simulink model "SOC_2023b"  (R2023b)
settings: Solver=VariableStepAuto  StartTime=0.0  StopTime=10.0  RelTol=1e-3
undefined symbols (not defined in the file or in its folder): Initial_SOC
(part 10 of 187)

== system SOC_2023b/SOC/Multi-Temperature SOC EKF5/Extended Kalman Filter - Multi Temperature EKF ==
  note: McMaster Automotive Research Centre - MARC
      | Electrical Engineer: Romulo Navega Vieira, M.Sc and MBA - PhD Candidate
  Current : Inport  Partition=on  PartitionDimension=1  PartitionWidth=1  PartitionOffset=0
  Voltage : Inport  Port=2  Partition=on  PartitionDimension=1  PartitionWidth=1  PartitionOffset=0
  Temperature : Inport  Port=3  Partition=on  PartitionDimension=1  PartitionWidth=1  PartitionOffset=0
  Constant{1..7} (7 alike) : Constant  Value=[0;0;0]
  Delay{1..2,5,7,9,11,13} (7 alike) : Delay  DelayLength=1  InitialConditionSource=Input port  InitialCondition=[0;0;0;Initial_SOC]
      settings: InputPortMap=u0,p4
  Delay{3..4,6,8,10,12,14} (7 alike) : Delay  DelayLength=1  InitialCondition=diag([1 1 1 0.01])
  EKF- 40deg C : MATLAB Function  TreatAsAtomicUnit=on
  EKF- 40deg C1 : MATLAB Function  Commented=on  TreatAsAtomicUnit=on
  EKF- 40deg C2 : MATLAB Function  Commented=on  TreatAsAtomicUnit=on
  EKF- 40deg C3 : MATLAB Function  Commented=on  TreatAsAtomicUnit=on
  EKF- 40deg C4 : MATLAB Function  Commented=on  TreatAsAtomicUnit=on
  EKF- 40deg C5 : MATLAB Function  Commented=on  TreatAsAtomicUnit=on
  EKF- 40deg C6 : MATLAB Function  Commented=on  TreatAsAtomicUnit=on
  For Each : ForEach  DisableCoverage=on
  MATLAB Function : MATLAB Function  TreatAsAtomicUnit=on
  MATLAB Function1 : MATLAB Function  TreatAsAtomicUnit=on
  MATLAB Function2 : MATLAB Function  TreatAsAtomicUnit=on
  MATLAB Function3 : MATLAB Function  TreatAsAtomicUnit=on
  MATLAB Function4 : MATLAB Function  TreatAsAtomicUnit=on
  MATLAB Function5 : MATLAB Function  TreatAsAtomicUnit=on
  MATLAB Function6 : MATLAB Function  TreatAsAtomicUnit=on
  MATLAB Function7 : MATLAB Function  TreatAsAtomicUnit=on
  Vector Concatenate{,1..6} (7 alike) : Concatenate
  SOC : Outport  ConcatenationDimension=1
  wires:
  Voltage/1 -> EKF- 40deg C1/2(Voltage), EKF- 40deg C2/2(Voltage), EKF- 40deg C3/2(Voltage), EKF- 40deg C4/2(Voltage), EKF- 40deg C5/2(Voltage), EKF- 40deg C6/2(Voltage), MATLAB Function7/1(Voltage), MATLAB Function6/1(Voltage), MATLAB Function5/1(Voltage), MATLAB Function4/1(Voltage), MATLAB Function3/1(Voltage), MATLAB Function2/1(Voltage), MATLAB Function/1(Voltage), EKF- 40deg C/2(Voltage)
  MATLAB Function/1(Initial_SOC) -> Vector Concatenate/2
  EKF- 40deg C4/1(zSOC) -> MATLAB Function1/6(SOC0)
  Delay14/1 -> EKF- 40deg C6/4(P)
  EKF- 40deg C3/2(xf) -> Delay7/1
  Vector Concatenate1/1 -> Delay1/2
  EKF- 40deg C6/1(zSOC) -> MATLAB Function1/8(SOCn20)
  EKF- 40deg C6/2(xf) -> Delay13/1
  Vector Concatenate3/1 -> Delay7/2
  MATLAB Function7/1(Initial_SOC) -> Vector Concatenate6/2
  Vector Concatenate2/1 -> Delay5/2
  EKF- 40deg C5/1(zSOC) -> MATLAB Function1/7(SOCn10)
  Constant7/1 -> Vector Concatenate6/1
  Vector Concatenate5/1 -> Delay11/2
  EKF- 40deg C1/3(P1) -> Delay4/1
  Constant6/1 -> Vector Concatenate5/1
  EKF- 40deg C6/3(P1) -> Delay14/1
  MATLAB Function1/1(SOCF) -> SOC/1
  Current/1 -> EKF- 40deg C6/1(Current), EKF- 40deg C5/1(Current), EKF- 40deg C4/1(Current), EKF- 40deg C3/1(Current), EKF- 40deg C2/1(Current), EKF- 40deg C1/1(Current), EKF- 40deg C/1(Current)
  EKF- 40deg C4/3(P1) -> Delay10/1
  Delay11/1 -> EKF- 40deg C5/3(x)
  Delay13/1 -> EKF- 40deg C6/3(x)
  MATLAB Function5/1(Initial_SOC) -> Vector Concatenate4/2
  MATLAB Function6/1(Initial_SOC) -> Vector Concatenate5/2
  EKF- 40deg C5/2(xf) -> Delay11/1
  Delay10/1 -> EKF- 40deg C4/4(P)
  EKF- 40deg C5/3(P1) -> Delay12/1
  Constant5/1 -> Vector Concatenate4/1
  Delay2/1 -> EKF- 40deg C/3(x)
  EKF- 40deg C4/2(xf) -> Delay9/1
  EKF- 40deg C3/1(zSOC) -> MATLAB Function1/5(SOC10)
  EKF- 40deg C2/3(P1) -> Delay6/1
  EKF- 40deg C2/1(zSOC) -> MATLAB Function1/4(SOC20)
  Vector Concatenate6/1 -> Delay13/2
  Constant4/1 -> Vector Concatenate3/1
  Temperature/1 -> MATLAB Function1/1(Temp)
  EKF- 40deg C2/2(xf) -> Delay5/1
  Delay7/1 -> EKF- 40deg C3/3(x)
  Vector Concatenate/1 -> Delay2/2
  Delay12/1 -> EKF- 40deg C5/4(P)
  Vector Concatenate4/1 -> Delay9/2
  EKF- 40deg C1/1(zSOC) -> MATLAB Function1/3(SOC30)
  Delay5/1 -> EKF- 40deg C2/3(x)
  MATLAB Function4/1(Initial_SOC) -> Vector Concatenate3/2
  Delay6/1 -> EKF- 40deg C2/4(P)
  EKF- 40deg C/2(xf) -> Delay2/1
  Delay3/1 -> EKF- 40deg C/4(P)
  EKF- 40deg C3/3(P1) -> Delay8/1
  Delay9/1 -> EKF- 40deg C4/3(x)
  Delay8/1 -> EKF- 40deg C3/4(P)
  Constant3/1 -> Vector Concatenate2/1
  MATLAB Function2/1(Initial_SOC) -> Vector Concatenate1/2
  EKF- 40deg C/1(zSOC) -> MATLAB Function1/2(SOC40)
  MATLAB Function3/1(Initial_SOC) -> Vector Concatenate2/2
  Constant2/1 -> Vector Concatenate1/1
  Constant1/1 -> Vector Concatenate/1
  Delay4/1 -> EKF- 40deg C1/4(P)
  EKF- 40deg C/3(P1) -> Delay3/1
  Delay1/1 -> EKF- 40deg C1/3(x)
  EKF- 40deg C1/2(xf) -> Delay1/1

== system SOC_2023b/SOC/Multi-Temperature SOC EKF50/Extended Kalman Filter - Multi Temperature EKF ==
  note: McMaster Automotive Research Centre - MARC
      | Electrical Engineer: Romulo Navega Vieira, M.Sc and MBA - PhD Candidate
  Current : Inport  Partition=on  PartitionDimension=1  PartitionWidth=1  PartitionOffset=0
  Voltage : Inport  Port=2  Partition=on  PartitionDimension=1  PartitionWidth=1  PartitionOffset=0
  Temperature : Inport  Port=3  Partition=on  PartitionDimension=1  PartitionWidth=1  PartitionOffset=0
  Constant{1..7} (7 alike) : Constant  Value=[0;0;0]
  Delay{1..2,5,7,9,11,13} (7 alike) : Delay  DelayLength=1  InitialConditionSource=Input port  InitialCondition=[0;0;0;Initial_SOC]
      settings: InputPortMap=u0,p4
  Delay{3..4,6,8,10,12,14} (7 alike) : Delay  DelayLength=1  InitialCondition=diag([1 1 1 0.01])
  EKF- 40deg C : MATLAB Function  TreatAsAtomicUnit=on
  EKF- 40deg C1 : MATLAB Function  Commented=on  TreatAsAtomicUnit=on
  EKF- 40deg C2 : MATLAB Function  Commented=on  TreatAsAtomicUnit=on
  EKF- 40deg C3 : MATLAB Function  Commented=on  TreatAsAtomicUnit=on
  EKF- 40deg C4 : MATLAB Function  Commented=on  TreatAsAtomicUnit=on
  EKF- 40deg C5 : MATLAB Function  Commented=on  TreatAsAtomicUnit=on
  EKF- 40deg C6 : MATLAB Function  Commented=on  TreatAsAtomicUnit=on
  For Each : ForEach  DisableCoverage=on
  MATLAB Function : MATLAB Function  TreatAsAtomicUnit=on
  MATLAB Function1 : MATLAB Function  TreatAsAtomicUnit=on
  MATLAB Function2 : MATLAB Function  TreatAsAtomicUnit=on
  MATLAB Function3 : MATLAB Function  TreatAsAtomicUnit=on
  MATLAB Function4 : MATLAB Function  TreatAsAtomicUnit=on
  MATLAB Function5 : MATLAB Function  TreatAsAtomicUnit=on
  MATLAB Function6 : MATLAB Function  TreatAsAtomicUnit=on
  MATLAB Function7 : MATLAB Function  TreatAsAtomicUnit=on
  Vector Concatenate{,1..6} (7 alike) : Concatenate
  SOC : Outport  ConcatenationDimension=1
  wires:
  Voltage/1 -> EKF- 40deg C1/2(Voltage), EKF- 40deg C2/2(Voltage), EKF- 40deg C3/2(Voltage), EKF- 40deg C4/2(Voltage), EKF- 40deg C5/2(Voltage), EKF- 40deg C6/2(Voltage), MATLAB Function7/1(Voltage), MATLAB Function6/1(Voltage), MATLAB Function5/1(Voltage), MATLAB Function4/1(Voltage), MATLAB Function3/1(Voltage), MATLAB Function2/1(Voltage), MATLAB Function/1(Voltage), EKF- 40deg C/2(Voltage)
  MATLAB Function/1(Initial_SOC) -> Vector Concatenate/2
  EKF- 40deg C4/1(zSOC) -> MATLAB Function1/6(SOC0)
  Delay14/1 -> EKF- 40deg C6/4(P)
  EKF- 40deg C3/2(xf) -> Delay7/1
  Vector Concatenate1/1 -> Delay1/2
  EKF- 40deg C6/1(zSOC) -> MATLAB Function1/8(SOCn20)
  EKF- 40deg C6/2(xf) -> Delay13/1
  Vector Concatenate3/1 -> Delay7/2
  MATLAB Function7/1(Initial_SOC) -> Vector Concatenate6/2
  Vector Concatenate2/1 -> Delay5/2
  EKF- 40deg C5/1(zSOC) -> MATLAB Function1/7(SOCn10)
  Constant7/1 -> Vector Concatenate6/1
  Vector Concatenate5/1 -> Delay11/2
  EKF- 40deg C1/3(P1) -> Delay4/1
  Constant6/1 -> Vector Concatenate5/1
  EKF- 40deg C6/3(P1) -> Delay14/1
  MATLAB Function1/1(SOCF) -> SOC/1
  Current/1 -> EKF- 40deg C6/1(Current), EKF- 40deg C5/1(Current), EKF- 40deg C4/1(Current), EKF- 40deg C3/1(Current), EKF- 40deg C2/1(Current), EKF- 40deg C1/1(Current), EKF- 40deg C/1(Current)
  EKF- 40deg C4/3(P1) -> Delay10/1
  Delay11/1 -> EKF- 40deg C5/3(x)
  Delay13/1 -> EKF- 40deg C6/3(x)
  MATLAB Function5/1(Initial_SOC) -> Vector Concatenate4/2
  MATLAB Function6/1(Initial_SOC) -> Vector Concatenate5/2
  EKF- 40deg C5/2(xf) -> Delay11/1
  Delay10/1 -> EKF- 40deg C4/4(P)
  EKF- 40deg C5/3(P1) -> Delay12/1
  Constant5/1 -> Vector Concatenate4/1
  Delay2/1 -> EKF- 40deg C/3(x)
  EKF- 40deg C4/2(xf) -> Delay9/1
  EKF- 40deg C3/1(zSOC) -> MATLAB Function1/5(SOC10)
  EKF- 40deg C2/3(P1) -> Delay6/1
  EKF- 40deg C2/1(zSOC) -> MATLAB Function1/4(SOC20)
  Vector Concatenate6/1 -> Delay13/2
  Constant4/1 -> Vector Concatenate3/1
  Temperature/1 -> MATLAB Function1/1(Temp)
  EKF- 40deg C2/2(xf) -> Delay5/1
  Delay7/1 -> EKF- 40deg C3/3(x)
  Vector Concatenate/1 -> Delay2/2
  Delay12/1 -> EKF- 40deg C5/4(P)
  Vector Concatenate4/1 -> Delay9/2
  EKF- 40deg C1/1(zSOC) -> MATLAB Function1/3(SOC30)
  Delay5/1 -> EKF- 40deg C2/3(x)
  MATLAB Function4/1(Initial_SOC) -> Vector Concatenate3/2
  Delay6/1 -> EKF- 40deg C2/4(P)
  EKF- 40deg C/2(xf) -> Delay2/1
  Delay3/1 -> EKF- 40deg C/4(P)
  EKF- 40deg C3/3(P1) -> Delay8/1
  Delay9/1 -> EKF- 40deg C4/3(x)
  Delay8/1 -> EKF- 40deg C3/4(P)
  Constant3/1 -> Vector Concatenate2/1
  MATLAB Function2/1(Initial_SOC) -> Vector Concatenate1/2
  EKF- 40deg C/1(zSOC) -> MATLAB Function1/2(SOC40)
  MATLAB Function3/1(Initial_SOC) -> Vector Concatenate2/2
  Constant2/1 -> Vector Concatenate1/1
  Constant1/1 -> Vector Concatenate/1
  Delay4/1 -> EKF- 40deg C1/4(P)
  EKF- 40deg C/3(P1) -> Delay3/1
  Delay1/1 -> EKF- 40deg C1/3(x)
  EKF- 40deg C1/2(xf) -> Delay1/1

== system SOC_2023b/SOC/Multi-Temperature SOC EKF51/Extended Kalman Filter - Multi Temperature EKF ==
  note: McMaster Automotive Research Centre - MARC
      | Electrical Engineer: Romulo Navega Vieira, M.Sc and MBA - PhD Candidate
  Current : Inport  Partition=on  PartitionDimension=1  PartitionWidth=1  PartitionOffset=0
  Voltage : Inport  Port=2  Partition=on  PartitionDimension=1  PartitionWidth=1  PartitionOffset=0
  Temperature : Inport  Port=3  Partition=on  PartitionDimension=1  PartitionWidth=1  PartitionOffset=0
  Constant{1..7} (7 alike) : Constant  Value=[0;0;0]
  Delay{1..2,5,7,9,11,13} (7 alike) : Delay  DelayLength=1  InitialConditionSource=Input port  InitialCondition=[0;0;0;Initial_SOC]
      settings: InputPortMap=u0,p4
  Delay{3..4,6,8,10,12,14} (7 alike) : Delay  DelayLength=1  InitialCondition=diag([1 1 1 0.01])
  EKF- 40deg C : MATLAB Function  TreatAsAtomicUnit=on
  EKF- 40deg C1 : MATLAB Function  Commented=on  TreatAsAtomicUnit=on
  EKF- 40deg C2 : MATLAB Function  Commented=on  TreatAsAtomicUnit=on
  EKF- 40deg C3 : MATLAB Function  Commented=on  TreatAsAtomicUnit=on
  EKF- 40deg C4 : MATLAB Function  Commented=on  TreatAsAtomicUnit=on
  EKF- 40deg C5 : MATLAB Function  Commented=on  TreatAsAtomicUnit=on
  EKF- 40deg C6 : MATLAB Function  Commented=on  TreatAsAtomicUnit=on
  For Each : ForEach  DisableCoverage=on
  MATLAB Function : MATLAB Function  TreatAsAtomicUnit=on
  MATLAB Function1 : MATLAB Function  TreatAsAtomicUnit=on
  MATLAB Function2 : MATLAB Function  TreatAsAtomicUnit=on
  MATLAB Function3 : MATLAB Function  TreatAsAtomicUnit=on
  MATLAB Function4 : MATLAB Function  TreatAsAtomicUnit=on
  MATLAB Function5 : MATLAB Function  TreatAsAtomicUnit=on
  MATLAB Function6 : MATLAB Function  TreatAsAtomicUnit=on
  MATLAB Function7 : MATLAB Function  TreatAsAtomicUnit=on
  Vector Concatenate{,1..6} (7 alike) : Concatenate
  SOC : Outport  ConcatenationDimension=1
  wires:
  Voltage/1 -> EKF- 40deg C1/2(Voltage), EKF- 40deg C2/2(Voltage), EKF- 40deg C3/2(Voltage), EKF- 40deg C4/2(Voltage), EKF- 40deg C5/2(Voltage), EKF- 40deg C6/2(Voltage), MATLAB Function7/1(Voltage), MATLAB Function6/1(Voltage), MATLAB Function5/1(Voltage), MATLAB Function4/1(Voltage), MATLAB Function3/1(Voltage), MATLAB Function2/1(Voltage), MATLAB Function/1(Voltage), EKF- 40deg C/2(Voltage)
  MATLAB Function/1(Initial_SOC) -> Vector Concatenate/2
  EKF- 40deg C4/1(zSOC) -> MATLAB Function1/6(SOC0)
  Delay14/1 -> EKF- 40deg C6/4(P)
  EKF- 40deg C3/2(xf) -> Delay7/1
  Vector Concatenate1/1 -> Delay1/2
  EKF- 40deg C6/1(zSOC) -> MATLAB Function1/8(SOCn20)
  EKF- 40deg C6/2(xf) -> Delay13/1
  Vector Concatenate3/1 -> Delay7/2
  MATLAB Function7/1(Initial_SOC) -> Vector Concatenate6/2
  Vector Concatenate2/1 -> Delay5/2
  EKF- 40deg C5/1(zSOC) -> MATLAB Function1/7(SOCn10)
  Constant7/1 -> Vector Concatenate6/1
  Vector Concatenate5/1 -> Delay11/2
  EKF- 40deg C1/3(P1) -> Delay4/1
  Constant6/1 -> Vector Concatenate5/1
  EKF- 40deg C6/3(P1) -> Delay14/1
  MATLAB Function1/1(SOCF) -> SOC/1
  Current/1 -> EKF- 40deg C6/1(Current), EKF- 40deg C5/1(Current), EKF- 40deg C4/1(Current), EKF- 40deg C3/1(Current), EKF- 40deg C2/1(Current), EKF- 40deg C1/1(Current), EKF- 40deg C/1(Current)
  EKF- 40deg C4/3(P1) -> Delay10/1
  Delay11/1 -> EKF- 40deg C5/3(x)
  Delay13/1 -> EKF- 40deg C6/3(x)
  MATLAB Function5/1(Initial_SOC) -> Vector Concatenate4/2
  MATLAB Function6/1(Initial_SOC) -> Vector Concatenate5/2
  EKF- 40deg C5/2(xf) -> Delay11/1
  Delay10/1 -> EKF- 40deg C4/4(P)
  EKF- 40deg C5/3(P1) -> Delay12/1
  Constant5/1 -> Vector Concatenate4/1
  Delay2/1 -> EKF- 40deg C/3(x)
  EKF- 40deg C4/2(xf) -> Delay9/1
  EKF- 40deg C3/1(zSOC) -> MATLAB Function1/5(SOC10)
  EKF- 40deg C2/3(P1) -> Delay6/1
  EKF- 40deg C2/1(zSOC) -> MATLAB Function1/4(SOC20)
  Vector Concatenate6/1 -> Delay13/2
  Constant4/1 -> Vector Concatenate3/1
  Temperature/1 -> MATLAB Function1/1(Temp)
  EKF- 40deg C2/2(xf) -> Delay5/1
  Delay7/1 -> EKF- 40deg C3/3(x)
  Vector Concatenate/1 -> Delay2/2
  Delay12/1 -> EKF- 40deg C5/4(P)
  Vector Concatenate4/1 -> Delay9/2
  EKF- 40deg C1/1(zSOC) -> MATLAB Function1/3(SOC30)
  Delay5/1 -> EKF- 40deg C2/3(x)
  MATLAB Function4/1(Initial_SOC) -> Vector Concatenate3/2
  Delay6/1 -> EKF- 40deg C2/4(P)
  EKF- 40deg C/2(xf) -> Delay2/1
  Delay3/1 -> EKF- 40deg C/4(P)
  EKF- 40deg C3/3(P1) -> Delay8/1
  Delay9/1 -> EKF- 40deg C4/3(x)
  Delay8/1 -> EKF- 40deg C3/4(P)
  Constant3/1 -> Vector Concatenate2/1
  MATLAB Function2/1(Initial_SOC) -> Vector Concatenate1/2
  EKF- 40deg C/1(zSOC) -> MATLAB Function1/2(SOC40)
  MATLAB Function3/1(Initial_SOC) -> Vector Concatenate2/2
  Constant2/1 -> Vector Concatenate1/1
  Constant1/1 -> Vector Concatenate/1
  Delay4/1 -> EKF- 40deg C1/4(P)
  EKF- 40deg C/3(P1) -> Delay3/1
  Delay1/1 -> EKF- 40deg C1/3(x)
  EKF- 40deg C1/2(xf) -> Delay1/1

== system SOC_2023b/SOC/Multi-Temperature SOC EKF52/Extended Kalman Filter - Multi Temperature EKF ==
  note: McMaster Automotive Research Centre - MARC
      | Electrical Engineer: Romulo Navega Vieira, M.Sc and MBA - PhD Candidate
  Current : Inport  Partition=on  PartitionDimension=1  PartitionWidth=1  PartitionOffset=0
  Voltage : Inport  Port=2  Partition=on  PartitionDimension=1  PartitionWidth=1  PartitionOffset=0
  Temperature : Inport  Port=3  Partition=on  PartitionDimension=1  PartitionWidth=1  PartitionOffset=0
  Constant{1..7} (7 alike) : Constant  Value=[0;0;0]
  Delay{1..2,5,7,9,11,13} (7 alike) : Delay  DelayLength=1  InitialConditionSource=Input port  InitialCondition=[0;0;0;Initial_SOC]
      settings: InputPortMap=u0,p4
  Delay{3..4,6,8,10,12,14} (7 alike) : Delay  DelayLength=1  InitialCondition=diag([1 1 1 0.01])
  EKF- 40deg C : MATLAB Function  TreatAsAtomicUnit=on
  EKF- 40deg C1 : MATLAB Function  Commented=on  TreatAsAtomicUnit=on
  EKF- 40deg C2 : MATLAB Function  Commented=on  TreatAsAtomicUnit=on
  EKF- 40deg C3 : MATLAB Function  Commented=on  TreatAsAtomicUnit=on
  EKF- 40deg C4 : MATLAB Function  Commented=on  TreatAsAtomicUnit=on
  EKF- 40deg C5 : MATLAB Function  Commented=on  TreatAsAtomicUnit=on
  EKF- 40deg C6 : MATLAB Function  Commented=on  TreatAsAtomicUnit=on
  For Each : ForEach  DisableCoverage=on
  MATLAB Function : MATLAB Function  TreatAsAtomicUnit=on
  MATLAB Function1 : MATLAB Function  TreatAsAtomicUnit=on
  MATLAB Function2 : MATLAB Function  TreatAsAtomicUnit=on
  MATLAB Function3 : MATLAB Function  TreatAsAtomicUnit=on
  MATLAB Function4 : MATLAB Function  TreatAsAtomicUnit=on
  MATLAB Function5 : MATLAB Function  TreatAsAtomicUnit=on
  MATLAB Function6 : MATLAB Function  TreatAsAtomicUnit=on
  MATLAB Function7 : MATLAB Function  TreatAsAtomicUnit=on
  Vector Concatenate{,1..6} (7 alike) : Concatenate
  SOC : Outport  ConcatenationDimension=1
  wires:
  Voltage/1 -> EKF- 40deg C1/2(Voltage), EKF- 40deg C2/2(Voltage), EKF- 40deg C3/2(Voltage), EKF- 40deg C4/2(Voltage), EKF- 40deg C5/2(Voltage), EKF- 40deg C6/2(Voltage), MATLAB Function7/1(Voltage), MATLAB Function6/1(Voltage), MATLAB Function5/1(Voltage), MATLAB Function4/1(Voltage), MATLAB Function3/1(Voltage), MATLAB Function2/1(Voltage), MATLAB Function/1(Voltage), EKF- 40deg C/2(Voltage)
  MATLAB Function/1(Initial_SOC) -> Vector Concatenate/2
  EKF- 40deg C4/1(zSOC) -> MATLAB Function1/6(SOC0)
  Delay14/1 -> EKF- 40deg C6/4(P)
  EKF- 40deg C3/2(xf) -> Delay7/1
  Vector Concatenate1/1 -> Delay1/2
  EKF- 40deg C6/1(zSOC) -> MATLAB Function1/8(SOCn20)
  EKF- 40deg C6/2(xf) -> Delay13/1
  Vector Concatenate3/1 -> Delay7/2
  MATLAB Function7/1(Initial_SOC) -> Vector Concatenate6/2
  Vector Concatenate2/1 -> Delay5/2
  EKF- 40deg C5/1(zSOC) -> MATLAB Function1/7(SOCn10)
  Constant7/1 -> Vector Concatenate6/1
  Vector Concatenate5/1 -> Delay11/2
  EKF- 40deg C1/3(P1) -> Delay4/1
  Constant6/1 -> Vector Concatenate5/1
  EKF- 40deg C6/3(P1) -> Delay14/1
  MATLAB Function1/1(SOCF) -> SOC/1
  Current/1 -> EKF- 40deg C6/1(Current), EKF- 40deg C5/1(Current), EKF- 40deg C4/1(Current), EKF- 40deg C3/1(Current), EKF- 40deg C2/1(Current), EKF- 40deg C1/1(Current), EKF- 40deg C/1(Current)
  EKF- 40deg C4/3(P1) -> Delay10/1
  Delay11/1 -> EKF- 40deg C5/3(x)
  Delay13/1 -> EKF- 40deg C6/3(x)
  MATLAB Function5/1(Initial_SOC) -> Vector Concatenate4/2
  MATLAB Function6/1(Initial_SOC) -> Vector Concatenate5/2
  EKF- 40deg C5/2(xf) -> Delay11/1
  Delay10/1 -> EKF- 40deg C4/4(P)
  EKF- 40deg C5/3(P1) -> Delay12/1
  Constant5/1 -> Vector Concatenate4/1
  Delay2/1 -> EKF- 40deg C/3(x)
  EKF- 40deg C4/2(xf) -> Delay9/1
  EKF- 40deg C3/1(zSOC) -> MATLAB Function1/5(SOC10)
  EKF- 40deg C2/3(P1) -> Delay6/1
  EKF- 40deg C2/1(zSOC) -> MATLAB Function1/4(SOC20)
  Vector Concatenate6/1 -> Delay13/2
  Constant4/1 -> Vector Concatenate3/1
  Temperature/1 -> MATLAB Function1/1(Temp)
  EKF- 40deg C2/2(xf) -> Delay5/1
  Delay7/1 -> EKF- 40deg C3/3(x)
  Vector Concatenate/1 -> Delay2/2
  Delay12/1 -> EKF- 40deg C5/4(P)
  Vector Concatenate4/1 -> Delay9/2
  EKF- 40deg C1/1(zSOC) -> MATLAB Function1/3(SOC30)
  Delay5/1 -> EKF- 40deg C2/3(x)
  MATLAB Function4/1(Initial_SOC) -> Vector Concatenate3/2
  Delay6/1 -> EKF- 40deg C2/4(P)
  EKF- 40deg C/2(xf) -> Delay2/1
  Delay3/1 -> EKF- 40deg C/4(P)
  EKF- 40deg C3/3(P1) -> Delay8/1
  Delay9/1 -> EKF- 40deg C4/3(x)
  Delay8/1 -> EKF- 40deg C3/4(P)
  Constant3/1 -> Vector Concatenate2/1
  MATLAB Function2/1(Initial_SOC) -> Vector Concatenate1/2
  EKF- 40deg C/1(zSOC) -> MATLAB Function1/2(SOC40)
  MATLAB Function3/1(Initial_SOC) -> Vector Concatenate2/2
  Constant2/1 -> Vector Concatenate1/1
  Constant1/1 -> Vector Concatenate/1
  Delay4/1 -> EKF- 40deg C1/4(P)
  EKF- 40deg C/3(P1) -> Delay3/1
  Delay1/1 -> EKF- 40deg C1/3(x)
  EKF- 40deg C1/2(xf) -> Delay1/1

== system SOC_2023b/SOC/Multi-Temperature SOC EKF53/Extended Kalman Filter - Multi Temperature EKF ==
  note: McMaster Automotive Research Centre - MARC
      | Electrical Engineer: Romulo Navega Vieira, M.Sc and MBA - PhD Candidate
  Current : Inport  Partition=on  PartitionDimension=1  PartitionWidth=1  PartitionOffset=0
  Voltage : Inport  Port=2  Partition=on  PartitionDimension=1  PartitionWidth=1  PartitionOffset=0
  Temperature : Inport  Port=3  Partition=on  PartitionDimension=1  PartitionWidth=1  PartitionOffset=0
  Constant{1..7} (7 alike) : Constant  Value=[0;0;0]
  Delay{1..2,5,7,9,11,13} (7 alike) : Delay  DelayLength=1  InitialConditionSource=Input port  InitialCondition=[0;0;0;Initial_SOC]
      settings: InputPortMap=u0,p4
  Delay{3..4,6,8,10,12,14} (7 alike) : Delay  DelayLength=1  InitialCondition=diag([1 1 1 0.01])
  EKF- 40deg C : MATLAB Function  TreatAsAtomicUnit=on
  EKF- 40deg C1 : MATLAB Function  Commented=on  TreatAsAtomicUnit=on
  EKF- 40deg C2 : MATLAB Function  Commented=on  TreatAsAtomicUnit=on
  EKF- 40deg C3 : MATLAB Function  Commented=on  TreatAsAtomicUnit=on
  EKF- 40deg C4 : MATLAB Function  Commented=on  TreatAsAtomicUnit=on
  EKF- 40deg C5 : MATLAB Function  Commented=on  TreatAsAtomicUnit=on
  EKF- 40deg C6 : MATLAB Function  Commented=on  TreatAsAtomicUnit=on
  For Each : ForEach  DisableCoverage=on
  MATLAB Function : MATLAB Function  TreatAsAtomicUnit=on
  MATLAB Function1 : MATLAB Function  TreatAsAtomicUnit=on
  MATLAB Function2 : MATLAB Function  TreatAsAtomicUnit=on
  MATLAB Function3 : MATLAB Function  TreatAsAtomicUnit=on
  MATLAB Function4 : MATLAB Function  TreatAsAtomicUnit=on
  MATLAB Function5 : MATLAB Function  TreatAsAtomicUnit=on
  MATLAB Function6 : MATLAB Function  TreatAsAtomicUnit=on
  MATLAB Function7 : MATLAB Function  TreatAsAtomicUnit=on
  Vector Concatenate{,1..6} (7 alike) : Concatenate
  SOC : Outport  ConcatenationDimension=1
  wires:
  Voltage/1 -> EKF- 40deg C1/2(Voltage), EKF- 40deg C2/2(Voltage), EKF- 40deg C3/2(Voltage), EKF- 40deg C4/2(Voltage), EKF- 40deg C5/2(Voltage), EKF- 40deg C6/2(Voltage), MATLAB Function7/1(Voltage), MATLAB Function6/1(Voltage), MATLAB Function5/1(Voltage), MATLAB Function4/1(Voltage), MATLAB Function3/1(Voltage), MATLAB Function2/1(Voltage), MATLAB Function/1(Voltage), EKF- 40deg C/2(Voltage)
  MATLAB Function/1(Initial_SOC) -> Vector Concatenate/2
  EKF- 40deg C4/1(zSOC) -> MATLAB Function1/6(SOC0)
  Delay14/1 -> EKF- 40deg C6/4(P)
  EKF- 40deg C3/2(xf) -> Delay7/1
  Vector Concatenate1/1 -> Delay1/2
  EKF- 40deg C6/1(zSOC) -> MATLAB Function1/8(SOCn20)
  EKF- 40deg C6/2(xf) -> Delay13/1
  Vector Concatenate3/1 -> Delay7/2
  MATLAB Function7/1(Initial_SOC) -> Vector Concatenate6/2
  Vector Concatenate2/1 -> Delay5/2
  EKF- 40deg C5/1(zSOC) -> MATLAB Function1/7(SOCn10)
  Constant7/1 -> Vector Concatenate6/1
  Vector Concatenate5/1 -> Delay11/2
  EKF- 40deg C1/3(P1) -> Delay4/1
  Constant6/1 -> Vector Concatenate5/1
  EKF- 40deg C6/3(P1) -> Delay14/1
  MATLAB Function1/1(SOCF) -> SOC/1
  Current/1 -> EKF- 40deg C6/1(Current), EKF- 40deg C5/1(Current), EKF- 40deg C4/1(Current), EKF- 40deg C3/1(Current), EKF- 40deg C2/1(Current), EKF- 40deg C1/1(Current), EKF- 40deg C/1(Current)
  EKF- 40deg C4/3(P1) -> Delay10/1
  Delay11/1 -> EKF- 40deg C5/3(x)
  Delay13/1 -> EKF- 40deg C6/3(x)
  MATLAB Function5/1(Initial_SOC) -> Vector Concatenate4/2
  MATLAB Function6/1(Initial_SOC) -> Vector Concatenate5/2
  EKF- 40deg C5/2(xf) -> Delay11/1
  Delay10/1 -> EKF- 40deg C4/4(P)
  EKF- 40deg C5/3(P1) -> Delay12/1
  Constant5/1 -> Vector Concatenate4/1
  Delay2/1 -> EKF- 40deg C/3(x)
  EKF- 40deg C4/2(xf) -> Delay9/1
  EKF- 40deg C3/1(zSOC) -> MATLAB Function1/5(SOC10)
  EKF- 40deg C2/3(P1) -> Delay6/1
  EKF- 40deg C2/1(zSOC) -> MATLAB Function1/4(SOC20)
  Vector Concatenate6/1 -> Delay13/2
  Constant4/1 -> Vector Concatenate3/1
  Temperature/1 -> MATLAB Function1/1(Temp)
  EKF- 40deg C2/2(xf) -> Delay5/1
  Delay7/1 -> EKF- 40deg C3/3(x)
  Vector Concatenate/1 -> Delay2/2
  Delay12/1 -> EKF- 40deg C5/4(P)
  Vector Concatenate4/1 -> Delay9/2
  EKF- 40deg C1/1(zSOC) -> MATLAB Function1/3(SOC30)
  Delay5/1 -> EKF- 40deg C2/3(x)
  MATLAB Function4/1(Initial_SOC) -> Vector Concatenate3/2
  Delay6/1 -> EKF- 40deg C2/4(P)
  EKF- 40deg C/2(xf) -> Delay2/1
  Delay3/1 -> EKF- 40deg C/4(P)
  EKF- 40deg C3/3(P1) -> Delay8/1
  Delay9/1 -> EKF- 40deg C4/3(x)
  Delay8/1 -> EKF- 40deg C3/4(P)
  Constant3/1 -> Vector Concatenate2/1
  MATLAB Function2/1(Initial_SOC) -> Vector Concatenate1/2
  EKF- 40deg C/1(zSOC) -> MATLAB Function1/2(SOC40)
  MATLAB Function3/1(Initial_SOC) -> Vector Concatenate2/2
  Constant2/1 -> Vector Concatenate1/1
  Constant1/1 -> Vector Concatenate/1
  Delay4/1 -> EKF- 40deg C1/4(P)
  EKF- 40deg C/3(P1) -> Delay3/1
  Delay1/1 -> EKF- 40deg C1/3(x)
  EKF- 40deg C1/2(xf) -> Delay1/1

== system SOC_2023b/SOC/Multi-Temperature SOC EKF54/Extended Kalman Filter - Multi Temperature EKF ==
  note: McMaster Automotive Research Centre - MARC
      | Electrical Engineer: Romulo Navega Vieira, M.Sc and MBA - PhD Candidate
  Current : Inport  Partition=on  PartitionDimension=1  PartitionWidth=1  PartitionOffset=0
  Voltage : Inport  Port=2  Partition=on  PartitionDimension=1  PartitionWidth=1  PartitionOffset=0
  Temperature : Inport  Port=3  Partition=on  PartitionDimension=1  PartitionWidth=1  PartitionOffset=0
  Constant{1..7} (7 alike) : Constant  Value=[0;0;0]
  Delay{1..2,5,7,9,11,13} (7 alike) : Delay  DelayLength=1  InitialConditionSource=Input port  InitialCondition=[0;0;0;Initial_SOC]
      settings: InputPortMap=u0,p4
  Delay{3..4,6,8,10,12,14} (7 alike) : Delay  DelayLength=1  InitialCondition=diag([1 1 1 0.01])
  EKF- 40deg C : MATLAB Function  TreatAsAtomicUnit=on
  EKF- 40deg C1 : MATLAB Function  Commented=on  TreatAsAtomicUnit=on
  EKF- 40deg C2 : MATLAB Function  Commented=on  TreatAsAtomicUnit=on
  EKF- 40deg C3 : MATLAB Function  Commented=on  TreatAsAtomicUnit=on
  EKF- 40deg C4 : MATLAB Function  Commented=on  TreatAsAtomicUnit=on
  EKF- 40deg C5 : MATLAB Function  Commented=on  TreatAsAtomicUnit=on
  EKF- 40deg C6 : MATLAB Function  Commented=on  TreatAsAtomicUnit=on
  For Each : ForEach  DisableCoverage=on
  MATLAB Function : MATLAB Function  TreatAsAtomicUnit=on
  MATLAB Function1 : MATLAB Function  TreatAsAtomicUnit=on
  MATLAB Function2 : MATLAB Function  TreatAsAtomicUnit=on
  MATLAB Function3 : MATLAB Function  TreatAsAtomicUnit=on
  MATLAB Function4 : MATLAB Function  TreatAsAtomicUnit=on
  MATLAB Function5 : MATLAB Function  TreatAsAtomicUnit=on
  MATLAB Function6 : MATLAB Function  TreatAsAtomicUnit=on
  MATLAB Function7 : MATLAB Function  TreatAsAtomicUnit=on
  Vector Concatenate{,1..6} (7 alike) : Concatenate
  SOC : Outport  ConcatenationDimension=1
  wires:
  Voltage/1 -> EKF- 40deg C1/2(Voltage), EKF- 40deg C2/2(Voltage), EKF- 40deg C3/2(Voltage), EKF- 40deg C4/2(Voltage), EKF- 40deg C5/2(Voltage), EKF- 40deg C6/2(Voltage), MATLAB Function7/1(Voltage), MATLAB Function6/1(Voltage), MATLAB Function5/1(Voltage), MATLAB Function4/1(Voltage), MATLAB Function3/1(Voltage), MATLAB Function2/1(Voltage), MATLAB Function/1(Voltage), EKF- 40deg C/2(Voltage)
  MATLAB Function/1(Initial_SOC) -> Vector Concatenate/2
  EKF- 40deg C4/1(zSOC) -> MATLAB Function1/6(SOC0)
  Delay14/1 -> EKF- 40deg C6/4(P)
  EKF- 40deg C3/2(xf) -> Delay7/1
  Vector Concatenate1/1 -> Delay1/2
  EKF- 40deg C6/1(zSOC) -> MATLAB Function1/8(SOCn20)
  EKF- 40deg C6/2(xf) -> Delay13/1
  Vector Concatenate3/1 -> Delay7/2
  MATLAB Function7/1(Initial_SOC) -> Vector Concatenate6/2
  Vector Concatenate2/1 -> Delay5/2
  EKF- 40deg C5/1(zSOC) -> MATLAB Function1/7(SOCn10)
  Constant7/1 -> Vector Concatenate6/1
  Vector Concatenate5/1 -> Delay11/2
  EKF- 40deg C1/3(P1) -> Delay4/1
  Constant6/1 -> Vector Concatenate5/1
  EKF- 40deg C6/3(P1) -> Delay14/1
  MATLAB Function1/1(SOCF) -> SOC/1
  Current/1 -> EKF- 40deg C6/1(Current), EKF- 40deg C5/1(Current), EKF- 40deg C4/1(Current), EKF- 40deg C3/1(Current), EKF- 40deg C2/1(Current), EKF- 40deg C1/1(Current), EKF- 40deg C/1(Current)
  EKF- 40deg C4/3(P1) -> Delay10/1
  Delay11/1 -> EKF- 40deg C5/3(x)
  Delay13/1 -> EKF- 40deg C6/3(x)
  MATLAB Function5/1(Initial_SOC) -> Vector Concatenate4/2
  MATLAB Function6/1(Initial_SOC) -> Vector Concatenate5/2
  EKF- 40deg C5/2(xf) -> Delay11/1
  Delay10/1 -> EKF- 40deg C4/4(P)
  EKF- 40deg C5/3(P1) -> Delay12/1
  Constant5/1 -> Vector Concatenate4/1
  Delay2/1 -> EKF- 40deg C/3(x)
  EKF- 40deg C4/2(xf) -> Delay9/1
  EKF- 40deg C3/1(zSOC) -> MATLAB Function1/5(SOC10)
  EKF- 40deg C2/3(P1) -> Delay6/1
  EKF- 40deg C2/1(zSOC) -> MATLAB Function1/4(SOC20)
  Vector Concatenate6/1 -> Delay13/2
  Constant4/1 -> Vector Concatenate3/1
  Temperature/1 -> MATLAB Function1/1(Temp)
  EKF- 40deg C2/2(xf) -> Delay5/1
  Delay7/1 -> EKF- 40deg C3/3(x)
  Vector Concatenate/1 -> Delay2/2
  Delay12/1 -> EKF- 40deg C5/4(P)
  Vector Concatenate4/1 -> Delay9/2
  EKF- 40deg C1/1(zSOC) -> MATLAB Function1/3(SOC30)
  Delay5/1 -> EKF- 40deg C2/3(x)
  MATLAB Function4/1(Initial_SOC) -> Vector Concatenate3/2
  Delay6/1 -> EKF- 40deg C2/4(P)
  EKF- 40deg C/2(xf) -> Delay2/1
  Delay3/1 -> EKF- 40deg C/4(P)
  EKF- 40deg C3/3(P1) -> Delay8/1
  Delay9/1 -> EKF- 40deg C4/3(x)
  Delay8/1 -> EKF- 40deg C3/4(P)
  Constant3/1 -> Vector Concatenate2/1
  MATLAB Function2/1(Initial_SOC) -> Vector Concatenate1/2
  EKF- 40deg C/1(zSOC) -> MATLAB Function1/2(SOC40)
  MATLAB Function3/1(Initial_SOC) -> Vector Concatenate2/2
  Constant2/1 -> Vector Concatenate1/1
  Constant1/1 -> Vector Concatenate/1
  Delay4/1 -> EKF- 40deg C1/4(P)
  EKF- 40deg C/3(P1) -> Delay3/1
  Delay1/1 -> EKF- 40deg C1/3(x)
  EKF- 40deg C1/2(xf) -> Delay1/1

== system SOC_2023b/SOC/Multi-Temperature SOC EKF55/Extended Kalman Filter - Multi Temperature EKF ==
  note: McMaster Automotive Research Centre - MARC
      | Electrical Engineer: Romulo Navega Vieira, M.Sc and MBA - PhD Candidate
  Current : Inport  Partition=on  PartitionDimension=1  PartitionWidth=1  PartitionOffset=0
  Voltage : Inport  Port=2  Partition=on  PartitionDimension=1  PartitionWidth=1  PartitionOffset=0
  Temperature : Inport  Port=3  Partition=on  PartitionDimension=1  PartitionWidth=1  PartitionOffset=0
  Constant{1..7} (7 alike) : Constant  Value=[0;0;0]
  Delay{1..2,5,7,9,11,13} (7 alike) : Delay  DelayLength=1  InitialConditionSource=Input port  InitialCondition=[0;0;0;Initial_SOC]
      settings: InputPortMap=u0,p4
  Delay{3..4,6,8,10,12,14} (7 alike) : Delay  DelayLength=1  InitialCondition=diag([1 1 1 0.01])
  EKF- 40deg C : MATLAB Function  TreatAsAtomicUnit=on
  EKF- 40deg C1 : MATLAB Function  Commented=on  TreatAsAtomicUnit=on
  EKF- 40deg C2 : MATLAB Function  Commented=on  TreatAsAtomicUnit=on
  EKF- 40deg C3 : MATLAB Function  Commented=on  TreatAsAtomicUnit=on
  EKF- 40deg C4 : MATLAB Function  Commented=on  TreatAsAtomicUnit=on
  EKF- 40deg C5 : MATLAB Function  Commented=on  TreatAsAtomicUnit=on
  EKF- 40deg C6 : MATLAB Function  Commented=on  TreatAsAtomicUnit=on
  For Each : ForEach  DisableCoverage=on
  MATLAB Function : MATLAB Function  TreatAsAtomicUnit=on
  MATLAB Function1 : MATLAB Function  TreatAsAtomicUnit=on
  MATLAB Function2 : MATLAB Function  TreatAsAtomicUnit=on
  MATLAB Function3 : MATLAB Function  TreatAsAtomicUnit=on
  MATLAB Function4 : MATLAB Function  TreatAsAtomicUnit=on
  MATLAB Function5 : MATLAB Function  TreatAsAtomicUnit=on
  MATLAB Function6 : MATLAB Function  TreatAsAtomicUnit=on
  MATLAB Function7 : MATLAB Function  TreatAsAtomicUnit=on
  Vector Concatenate{,1..6} (7 alike) : Concatenate
  SOC : Outport  ConcatenationDimension=1
  wires:
  Voltage/1 -> EKF- 40deg C1/2(Voltage), EKF- 40deg C2/2(Voltage), EKF- 40deg C3/2(Voltage), EKF- 40deg C4/2(Voltage), EKF- 40deg C5/2(Voltage), EKF- 40deg C6/2(Voltage), MATLAB Function7/1(Voltage), MATLAB Function6/1(Voltage), MATLAB Function5/1(Voltage), MATLAB Function4/1(Voltage), MATLAB Function3/1(Voltage), MATLAB Function2/1(Voltage), MATLAB Function/1(Voltage), EKF- 40deg C/2(Voltage)
  MATLAB Function/1(Initial_SOC) -> Vector Concatenate/2
  EKF- 40deg C4/1(zSOC) -> MATLAB Function1/6(SOC0)
  Delay14/1 -> EKF- 40deg C6/4(P)
  EKF- 40deg C3/2(xf) -> Delay7/1
  Vector Concatenate1/1 -> Delay1/2
  EKF- 40deg C6/1(zSOC) -> MATLAB Function1/8(SOCn20)
  EKF- 40deg C6/2(xf) -> Delay13/1
  Vector Concatenate3/1 -> Delay7/2
  MATLAB Function7/1(Initial_SOC) -> Vector Concatenate6/2
  Vector Concatenate2/1 -> Delay5/2
  EKF- 40deg C5/1(zSOC) -> MATLAB Function1/7(SOCn10)
  Constant7/1 -> Vector Concatenate6/1
  Vector Concatenate5/1 -> Delay11/2
  EKF- 40deg C1/3(P1) -> Delay4/1
  Constant6/1 -> Vector Concatenate5/1
  EKF- 40deg C6/3(P1) -> Delay14/1
  MATLAB Function1/1(SOCF) -> SOC/1
  Current/1 -> EKF- 40deg C6/1(Current), EKF- 40deg C5/1(Current), EKF- 40deg C4/1(Current), EKF- 40deg C3/1(Current), EKF- 40deg C2/1(Current), EKF- 40deg C1/1(Current), EKF- 40deg C/1(Current)
  EKF- 40deg C4/3(P1) -> Delay10/1
  Delay11/1 -> EKF- 40deg C5/3(x)
  Delay13/1 -> EKF- 40deg C6/3(x)
  MATLAB Function5/1(Initial_SOC) -> Vector Concatenate4/2
  MATLAB Function6/1(Initial_SOC) -> Vector Concatenate5/2
  EKF- 40deg C5/2(xf) -> Delay11/1
  Delay10/1 -> EKF- 40deg C4/4(P)
  EKF- 40deg C5/3(P1) -> Delay12/1
  Constant5/1 -> Vector Concatenate4/1
  Delay2/1 -> EKF- 40deg C/3(x)
  EKF- 40deg C4/2(xf) -> Delay9/1
  EKF- 40deg C3/1(zSOC) -> MATLAB Function1/5(SOC10)
  EKF- 40deg C2/3(P1) -> Delay6/1
  EKF- 40deg C2/1(zSOC) -> MATLAB Function1/4(SOC20)
  Vector Concatenate6/1 -> Delay13/2
  Constant4/1 -> Vector Concatenate3/1
  Temperature/1 -> MATLAB Function1/1(Temp)
  EKF- 40deg C2/2(xf) -> Delay5/1
  Delay7/1 -> EKF- 40deg C3/3(x)
  Vector Concatenate/1 -> Delay2/2
  Delay12/1 -> EKF- 40deg C5/4(P)
  Vector Concatenate4/1 -> Delay9/2
  EKF- 40deg C1/1(zSOC) -> MATLAB Function1/3(SOC30)
  Delay5/1 -> EKF- 40deg C2/3(x)
  MATLAB Function4/1(Initial_SOC) -> Vector Concatenate3/2
  Delay6/1 -> EKF- 40deg C2/4(P)
  EKF- 40deg C/2(xf) -> Delay2/1
  Delay3/1 -> EKF- 40deg C/4(P)
  EKF- 40deg C3/3(P1) -> Delay8/1
  Delay9/1 -> EKF- 40deg C4/3(x)
  Delay8/1 -> EKF- 40deg C3/4(P)
  Constant3/1 -> Vector Concatenate2/1
  MATLAB Function2/1(Initial_SOC) -> Vector Concatenate1/2
  EKF- 40deg C/1(zSOC) -> MATLAB Function1/2(SOC40)
  MATLAB Function3/1(Initial_SOC) -> Vector Concatenate2/2
  Constant2/1 -> Vector Concatenate1/1
  Constant1/1 -> Vector Concatenate/1
  Delay4/1 -> EKF- 40deg C1/4(P)
  EKF- 40deg C/3(P1) -> Delay3/1
  Delay1/1 -> EKF- 40deg C1/3(x)
  EKF- 40deg C1/2(xf) -> Delay1/1

== system SOC_2023b/SOC/Multi-Temperature SOC EKF56/Extended Kalman Filter - Multi Temperature EKF ==
  note: McMaster Automotive Research Centre - MARC
      | Electrical Engineer: Romulo Navega Vieira, M.Sc and MBA - PhD Candidate
  Current : Inport  Partition=on  PartitionDimension=1  PartitionWidth=1  PartitionOffset=0
  Voltage : Inport  Port=2  Partition=on  PartitionDimension=1  PartitionWidth=1  PartitionOffset=0
  Temperature : Inport  Port=3  Partition=on  PartitionDimension=1  PartitionWidth=1  PartitionOffset=0
  Constant{1..7} (7 alike) : Constant  Value=[0;0;0]
  Delay{1..2,5,7,9,11,13} (7 alike) : Delay  DelayLength=1  InitialConditionSource=Input port  InitialCondition=[0;0;0;Initial_SOC]
      settings: InputPortMap=u0,p4
  Delay{3..4,6,8,10,12,14} (7 alike) : Delay  DelayLength=1  InitialCondition=diag([1 1 1 0.01])
  EKF- 40deg C : MATLAB Function  TreatAsAtomicUnit=on
  EKF- 40deg C1 : MATLAB Function  Commented=on  TreatAsAtomicUnit=on
  EKF- 40deg C2 : MATLAB Function  Commented=on  TreatAsAtomicUnit=on
  EKF- 40deg C3 : MATLAB Function  Commented=on  TreatAsAtomicUnit=on
  EKF- 40deg C4 : MATLAB Function  Commented=on  TreatAsAtomicUnit=on
  EKF- 40deg C5 : MATLAB Function  Commented=on  TreatAsAtomicUnit=on
  EKF- 40deg C6 : MATLAB Function  Commented=on  TreatAsAtomicUnit=on
  For Each : ForEach  DisableCoverage=on
  MATLAB Function : MATLAB Function  TreatAsAtomicUnit=on
  MATLAB Function1 : MATLAB Function  TreatAsAtomicUnit=on
  MATLAB Function2 : MATLAB Function  TreatAsAtomicUnit=on
  MATLAB Function3 : MATLAB Function  TreatAsAtomicUnit=on
  MATLAB Function4 : MATLAB Function  TreatAsAtomicUnit=on
  MATLAB Function5 : MATLAB Function  TreatAsAtomicUnit=on
  MATLAB Function6 : MATLAB Function  TreatAsAtomicUnit=on
  MATLAB Function7 : MATLAB Function  TreatAsAtomicUnit=on
  Vector Concatenate{,1..6} (7 alike) : Concatenate
  SOC : Outport  ConcatenationDimension=1
  wires:
  Voltage/1 -> EKF- 40deg C1/2(Voltage), EKF- 40deg C2/2(Voltage), EKF- 40deg C3/2(Voltage), EKF- 40deg C4/2(Voltage), EKF- 40deg C5/2(Voltage), EKF- 40deg C6/2(Voltage), MATLAB Function7/1(Voltage), MATLAB Function6/1(Voltage), MATLAB Function5/1(Voltage), MATLAB Function4/1(Voltage), MATLAB Function3/1(Voltage), MATLAB Function2/1(Voltage), MATLAB Function/1(Voltage), EKF- 40deg C/2(Voltage)
  MATLAB Function/1(Initial_SOC) -> Vector Concatenate/2
  EKF- 40deg C4/1(zSOC) -> MATLAB Function1/6(SOC0)
  Delay14/1 -> EKF- 40deg C6/4(P)
  EKF- 40deg C3/2(xf) -> Delay7/1
  Vector Concatenate1/1 -> Delay1/2
  EKF- 40deg C6/1(zSOC) -> MATLAB Function1/8(SOCn20)
  EKF- 40deg C6/2(xf) -> Delay13/1
  Vector Concatenate3/1 -> Delay7/2
  MATLAB Function7/1(Initial_SOC) -> Vector Concatenate6/2
  Vector Concatenate2/1 -> Delay5/2
  EKF- 40deg C5/1(zSOC) -> MATLAB Function1/7(SOCn10)
  Constant7/1 -> Vector Concatenate6/1
  Vector Concatenate5/1 -> Delay11/2
  EKF- 40deg C1/3(P1) -> Delay4/1
  Constant6/1 -> Vector Concatenate5/1
  EKF- 40deg C6/3(P1) -> Delay14/1
  MATLAB Function1/1(SOCF) -> SOC/1
  Current/1 -> EKF- 40deg C6/1(Current), EKF- 40deg C5/1(Current), EKF- 40deg C4/1(Current), EKF- 40deg C3/1(Current), EKF- 40deg C2/1(Current), EKF- 40deg C1/1(Current), EKF- 40deg C/1(Current)
  EKF- 40deg C4/3(P1) -> Delay10/1
  Delay11/1 -> EKF- 40deg C5/3(x)
  Delay13/1 -> EKF- 40deg C6/3(x)
  MATLAB Function5/1(Initial_SOC) -> Vector Concatenate4/2
  MATLAB Function6/1(Initial_SOC) -> Vector Concatenate5/2
  EKF- 40deg C5/2(xf) -> Delay11/1
  Delay10/1 -> EKF- 40deg C4/4(P)
  EKF- 40deg C5/3(P1) -> Delay12/1
  Constant5/1 -> Vector Concatenate4/1
  Delay2/1 -> EKF- 40deg C/3(x)
  EKF- 40deg C4/2(xf) -> Delay9/1
  EKF- 40deg C3/1(zSOC) -> MATLAB Function1/5(SOC10)
  EKF- 40deg C2/3(P1) -> Delay6/1
  EKF- 40deg C2/1(zSOC) -> MATLAB Function1/4(SOC20)
  Vector Concatenate6/1 -> Delay13/2
  Constant4/1 -> Vector Concatenate3/1
  Temperature/1 -> MATLAB Function1/1(Temp)
  EKF- 40deg C2/2(xf) -> Delay5/1
  Delay7/1 -> EKF- 40deg C3/3(x)
  Vector Concatenate/1 -> Delay2/2
  Delay12/1 -> EKF- 40deg C5/4(P)
  Vector Concatenate4/1 -> Delay9/2
  EKF- 40deg C1/1(zSOC) -> MATLAB Function1/3(SOC30)
  Delay5/1 -> EKF- 40deg C2/3(x)
  MATLAB Function4/1(Initial_SOC) -> Vector Concatenate3/2
  Delay6/1 -> EKF- 40deg C2/4(P)
  EKF- 40deg C/2(xf) -> Delay2/1
  Delay3/1 -> EKF- 40deg C/4(P)
  EKF- 40deg C3/3(P1) -> Delay8/1
  Delay9/1 -> EKF- 40deg C4/3(x)
  Delay8/1 -> EKF- 40deg C3/4(P)
  Constant3/1 -> Vector Concatenate2/1
  MATLAB Function2/1(Initial_SOC) -> Vector Concatenate1/2
  EKF- 40deg C/1(zSOC) -> MATLAB Function1/2(SOC40)
  MATLAB Function3/1(Initial_SOC) -> Vector Concatenate2/2
  Constant2/1 -> Vector Concatenate1/1
  Constant1/1 -> Vector Concatenate/1
  Delay4/1 -> EKF- 40deg C1/4(P)
  EKF- 40deg C/3(P1) -> Delay3/1
  Delay1/1 -> EKF- 40deg C1/3(x)
  EKF- 40deg C1/2(xf) -> Delay1/1

== system SOC_2023b/SOC/Multi-Temperature SOC EKF57/Extended Kalman Filter - Multi Temperature EKF ==
  note: McMaster Automotive Research Centre - MARC
      | Electrical Engineer: Romulo Navega Vieira, M.Sc and MBA - PhD Candidate
  Current : Inport  Partition=on  PartitionDimension=1  PartitionWidth=1  PartitionOffset=0
  Voltage : Inport  Port=2  Partition=on  PartitionDimension=1  PartitionWidth=1  PartitionOffset=0
  Temperature : Inport  Port=3  Partition=on  PartitionDimension=1  PartitionWidth=1  PartitionOffset=0
  Constant{1..7} (7 alike) : Constant  Value=[0;0;0]
  Delay{1..2,5,7,9,11,13} (7 alike) : Delay  DelayLength=1  InitialConditionSource=Input port  InitialCondition=[0;0;0;Initial_SOC]
      settings: InputPortMap=u0,p4
  Delay{3..4,6,8,10,12,14} (7 alike) : Delay  DelayLength=1  InitialCondition=diag([1 1 1 0.01])
  EKF- 40deg C : MATLAB Function  TreatAsAtomicUnit=on
  EKF- 40deg C1 : MATLAB Function  Commented=on  TreatAsAtomicUnit=on
  EKF- 40deg C2 : MATLAB Function  Commented=on  TreatAsAtomicUnit=on
  EKF- 40deg C3 : MATLAB Function  Commented=on  TreatAsAtomicUnit=on
  EKF- 40deg C4 : MATLAB Function  Commented=on  TreatAsAtomicUnit=on
  EKF- 40deg C5 : MATLAB Function  Commented=on  TreatAsAtomicUnit=on
  EKF- 40deg C6 : MATLAB Function  Commented=on  TreatAsAtomicUnit=on
  For Each : ForEach  DisableCoverage=on
  MATLAB Function : MATLAB Function  TreatAsAtomicUnit=on
  MATLAB Function1 : MATLAB Function  TreatAsAtomicUnit=on
  MATLAB Function2 : MATLAB Function  TreatAsAtomicUnit=on
  MATLAB Function3 : MATLAB Function  TreatAsAtomicUnit=on
  MATLAB Function4 : MATLAB Function  TreatAsAtomicUnit=on
  MATLAB Function5 : MATLAB Function  TreatAsAtomicUnit=on
  MATLAB Function6 : MATLAB Function  TreatAsAtomicUnit=on
  MATLAB Function7 : MATLAB Function  TreatAsAtomicUnit=on
  Vector Concatenate{,1..6} (7 alike) : Concatenate
  SOC : Outport  ConcatenationDimension=1
  wires:
  Voltage/1 -> EKF- 40deg C1/2(Voltage), EKF- 40deg C2/2(Voltage), EKF- 40deg C3/2(Voltage), EKF- 40deg C4/2(Voltage), EKF- 40deg C5/2(Voltage), EKF- 40deg C6/2(Voltage), MATLAB Function7/1(Voltage), MATLAB Function6/1(Voltage), MATLAB Function5/1(Voltage), MATLAB Function4/1(Voltage), MATLAB Function3/1(Voltage), MATLAB Function2/1(Voltage), MATLAB Function/1(Voltage), EKF- 40deg C/2(Voltage)
  MATLAB Function/1(Initial_SOC) -> Vector Concatenate/2
  EKF- 40deg C4/1(zSOC) -> MATLAB Function1/6(SOC0)
  Delay14/1 -> EKF- 40deg C6/4(P)
  EKF- 40deg C3/2(xf) -> Delay7/1
  Vector Concatenate1/1 -> Delay1/2
  EKF- 40deg C6/1(zSOC) -> MATLAB Function1/8(SOCn20)
  EKF- 40deg C6/2(xf) -> Delay13/1
  Vector Concatenate3/1 -> Delay7/2
  MATLAB Function7/1(Initial_SOC) -> Vector Concatenate6/2
  Vector Concatenate2/1 -> Delay5/2
  EKF- 40deg C5/1(zSOC) -> MATLAB Function1/7(SOCn10)
  Constant7/1 -> Vector Concatenate6/1
  Vector Concatenate5/1 -> Delay11/2
  EKF- 40deg C1/3(P1) -> Delay4/1
  Constant6/1 -> Vector Concatenate5/1
  EKF- 40deg C6/3(P1) -> Delay14/1
  MATLAB Function1/1(SOCF) -> SOC/1
  Current/1 -> EKF- 40deg C6/1(Current), EKF- 40deg C5/1(Current), EKF- 40deg C4/1(Current), EKF- 40deg C3/1(Current), EKF- 40deg C2/1(Current), EKF- 40deg C1/1(Current), EKF- 40deg C/1(Current)
  EKF- 40deg C4/3(P1) -> Delay10/1
  Delay11/1 -> EKF- 40deg C5/3(x)
  Delay13/1 -> EKF- 40deg C6/3(x)
  MATLAB Function5/1(Initial_SOC) -> Vector Concatenate4/2
  MATLAB Function6/1(Initial_SOC) -> Vector Concatenate5/2
  EKF- 40deg C5/2(xf) -> Delay11/1
  Delay10/1 -> EKF- 40deg C4/4(P)
  EKF- 40deg C5/3(P1) -> Delay12/1
  Constant5/1 -> Vector Concatenate4/1
  Delay2/1 -> EKF- 40deg C/3(x)
  EKF- 40deg C4/2(xf) -> Delay9/1
  EKF- 40deg C3/1(zSOC) -> MATLAB Function1/5(SOC10)
  EKF- 40deg C2/3(P1) -> Delay6/1
  EKF- 40deg C2/1(zSOC) -> MATLAB Function1/4(SOC20)
  Vector Concatenate6/1 -> Delay13/2
  Constant4/1 -> Vector Concatenate3/1
  Temperature/1 -> MATLAB Function1/1(Temp)
  EKF- 40deg C2/2(xf) -> Delay5/1
  Delay7/1 -> EKF- 40deg C3/3(x)
  Vector Concatenate/1 -> Delay2/2
  Delay12/1 -> EKF- 40deg C5/4(P)
  Vector Concatenate4/1 -> Delay9/2
  EKF- 40deg C1/1(zSOC) -> MATLAB Function1/3(SOC30)
  Delay5/1 -> EKF- 40deg C2/3(x)
  MATLAB Function4/1(Initial_SOC) -> Vector Concatenate3/2
  Delay6/1 -> EKF- 40deg C2/4(P)
  EKF- 40deg C/2(xf) -> Delay2/1
  Delay3/1 -> EKF- 40deg C/4(P)
  EKF- 40deg C3/3(P1) -> Delay8/1
  Delay9/1 -> EKF- 40deg C4/3(x)
  Delay8/1 -> EKF- 40deg C3/4(P)
  Constant3/1 -> Vector Concatenate2/1
  MATLAB Function2/1(Initial_SOC) -> Vector Concatenate1/2
  EKF- 40deg C/1(zSOC) -> MATLAB Function1/2(SOC40)
  MATLAB Function3/1(Initial_SOC) -> Vector Concatenate2/2
  Constant2/1 -> Vector Concatenate1/1
  Constant1/1 -> Vector Concatenate/1
  Delay4/1 -> EKF- 40deg C1/4(P)
  EKF- 40deg C/3(P1) -> Delay3/1
  Delay1/1 -> EKF- 40deg C1/3(x)
  EKF- 40deg C1/2(xf) -> Delay1/1

== system SOC_2023b/SOC/Multi-Temperature SOC EKF58/Extended Kalman Filter - Multi Temperature EKF ==
  note: McMaster Automotive Research Centre - MARC
      | Electrical Engineer: Romulo Navega Vieira, M.Sc and MBA - PhD Candidate
  Current : Inport  Partition=on  PartitionDimension=1  PartitionWidth=1  PartitionOffset=0
  Voltage : Inport  Port=2  Partition=on  PartitionDimension=1  PartitionWidth=1  PartitionOffset=0
  Temperature : Inport  Port=3  Partition=on  PartitionDimension=1  PartitionWidth=1  PartitionOffset=0
  Constant{1..7} (7 alike) : Constant  Value=[0;0;0]
  Delay{1..2,5,7,9,11,13} (7 alike) : Delay  DelayLength=1  InitialConditionSource=Input port  InitialCondition=[0;0;0;Initial_SOC]
      settings: InputPortMap=u0,p4
  Delay{3..4,6,8,10,12,14} (7 alike) : Delay  DelayLength=1  InitialCondition=diag([1 1 1 0.01])
  EKF- 40deg C : MATLAB Function  TreatAsAtomicUnit=on
  EKF- 40deg C1 : MATLAB Function  Commented=on  TreatAsAtomicUnit=on
  EKF- 40deg C2 : MATLAB Function  Commented=on  TreatAsAtomicUnit=on
  EKF- 40deg C3 : MATLAB Function  Commented=on  TreatAsAtomicUnit=on
  EKF- 40deg C4 : MATLAB Function  Commented=on  TreatAsAtomicUnit=on
  EKF- 40deg C5 : MATLAB Function  Commented=on  TreatAsAtomicUnit=on
  EKF- 40deg C6 : MATLAB Function  Commented=on  TreatAsAtomicUnit=on
  For Each : ForEach  DisableCoverage=on
  MATLAB Function : MATLAB Function  TreatAsAtomicUnit=on
  MATLAB Function1 : MATLAB Function  TreatAsAtomicUnit=on
  MATLAB Function2 : MATLAB Function  TreatAsAtomicUnit=on
  MATLAB Function3 : MATLAB Function  TreatAsAtomicUnit=on
  MATLAB Function4 : MATLAB Function  TreatAsAtomicUnit=on
  MATLAB Function5 : MATLAB Function  TreatAsAtomicUnit=on
  MATLAB Function6 : MATLAB Function  TreatAsAtomicUnit=on
  MATLAB Function7 : MATLAB Function  TreatAsAtomicUnit=on
  Vector Concatenate{,1..6} (7 alike) : Concatenate
  SOC : Outport  ConcatenationDimension=1
  wires:
  Voltage/1 -> EKF- 40deg C1/2(Voltage), EKF- 40deg C2/2(Voltage), EKF- 40deg C3/2(Voltage), EKF- 40deg C4/2(Voltage), EKF- 40deg C5/2(Voltage), EKF- 40deg C6/2(Voltage), MATLAB Function7/1(Voltage), MATLAB Function6/1(Voltage), MATLAB Function5/1(Voltage), MATLAB Function4/1(Voltage), MATLAB Function3/1(Voltage), MATLAB Function2/1(Voltage), MATLAB Function/1(Voltage), EKF- 40deg C/2(Voltage)
  MATLAB Function/1(Initial_SOC) -> Vector Concatenate/2
  EKF- 40deg C4/1(zSOC) -> MATLAB Function1/6(SOC0)
  Delay14/1 -> EKF- 40deg C6/4(P)
  EKF- 40deg C3/2(xf) -> Delay7/1
  Vector Concatenate1/1 -> Delay1/2
  EKF- 40deg C6/1(zSOC) -> MATLAB Function1/8(SOCn20)
  EKF- 40deg C6/2(xf) -> Delay13/1
  Vector Concatenate3/1 -> Delay7/2
  MATLAB Function7/1(Initial_SOC) -> Vector Concatenate6/2
  Vector Concatenate2/1 -> Delay5/2
  EKF- 40deg C5/1(zSOC) -> MATLAB Function1/7(SOCn10)
  Constant7/1 -> Vector Concatenate6/1
  Vector Concatenate5/1 -> Delay11/2
  EKF- 40deg C1/3(P1) -> Delay4/1
  Constant6/1 -> Vector Concatenate5/1
  EKF- 40deg C6/3(P1) -> Delay14/1
  MATLAB Function1/1(SOCF) -> SOC/1
  Current/1 -> EKF- 40deg C6/1(Current), EKF- 40deg C5/1(Current), EKF- 40deg C4/1(Current), EKF- 40deg C3/1(Current), EKF- 40deg C2/1(Current), EKF- 40deg C1/1(Current), EKF- 40deg C/1(Current)
  EKF- 40deg C4/3(P1) -> Delay10/1
  Delay11/1 -> EKF- 40deg C5/3(x)
  Delay13/1 -> EKF- 40deg C6/3(x)
  MATLAB Function5/1(Initial_SOC) -> Vector Concatenate4/2
  MATLAB Function6/1(Initial_SOC) -> Vector Concatenate5/2
  EKF- 40deg C5/2(xf) -> Delay11/1
  Delay10/1 -> EKF- 40deg C4/4(P)
  EKF- 40deg C5/3(P1) -> Delay12/1
  Constant5/1 -> Vector Concatenate4/1
  Delay2/1 -> EKF- 40deg C/3(x)
  EKF- 40deg C4/2(xf) -> Delay9/1
  EKF- 40deg C3/1(zSOC) -> MATLAB Function1/5(SOC10)
  EKF- 40deg C2/3(P1) -> Delay6/1
  EKF- 40deg C2/1(zSOC) -> MATLAB Function1/4(SOC20)
  Vector Concatenate6/1 -> Delay13/2
  Constant4/1 -> Vector Concatenate3/1
  Temperature/1 -> MATLAB Function1/1(Temp)
  EKF- 40deg C2/2(xf) -> Delay5/1
  Delay7/1 -> EKF- 40deg C3/3(x)
  Vector Concatenate/1 -> Delay2/2
  Delay12/1 -> EKF- 40deg C5/4(P)
  Vector Concatenate4/1 -> Delay9/2
  EKF- 40deg C1/1(zSOC) -> MATLAB Function1/3(SOC30)
  Delay5/1 -> EKF- 40deg C2/3(x)
  MATLAB Function4/1(Initial_SOC) -> Vector Concatenate3/2
  Delay6/1 -> EKF- 40deg C2/4(P)
  EKF- 40deg C/2(xf) -> Delay2/1
  Delay3/1 -> EKF- 40deg C/4(P)
  EKF- 40deg C3/3(P1) -> Delay8/1
  Delay9/1 -> EKF- 40deg C4/3(x)
  Delay8/1 -> EKF- 40deg C3/4(P)
  Constant3/1 -> Vector Concatenate2/1
  MATLAB Function2/1(Initial_SOC) -> Vector Concatenate1/2
  EKF- 40deg C/1(zSOC) -> MATLAB Function1/2(SOC40)
  MATLAB Function3/1(Initial_SOC) -> Vector Concatenate2/2
  Constant2/1 -> Vector Concatenate1/1
  Constant1/1 -> Vector Concatenate/1
  Delay4/1 -> EKF- 40deg C1/4(P)
  EKF- 40deg C/3(P1) -> Delay3/1
  Delay1/1 -> EKF- 40deg C1/3(x)
  EKF- 40deg C1/2(xf) -> Delay1/1
